simulink model "yolov9_orin_ipcam_detect"  (R2025b)
settings: Solver=FixedStepAuto  StartTime=0.0  StopTime=10.0  FixedStep=0.05  RelTol=1e-3
libraries: nvidiaAVlib x2
settings Model: Creator=The MathWorks, Inc.  LastModifiedBy=Dogukan.kilinc
settings SolverCC: AlgebraicLoopSolver=TrustRegion
settings OptimizationCC: EnhancedBackFolding=on  PassReuseOutputArgsAs=Individual arguments  ZeroExternalMemoryAtStartup=off  ZeroInternalMemoryAtStartup=off  InitFltsAndDblsToZero=on  MaxStackSize=64  OptimizeBlockOrder=speed  DifferentSizesBufferReuse=on  OptimizationCustomize=off  EfficientTunableParamExpr=on
settings DebuggingCC: ParameterTunabilityLossMsg=error  UnconnectedInputMsg=warning  UnconnectedOutputMsg=warning  UnconnectedLineMsg=warning
settings HardwareCC: ProdBitPerLong=64.0  ProdLargestAtomicInteger=LongLong  ProdLargestAtomicFloat=Double  ProdLongLongMode=on  ProdHWDeviceType=ARM Compatible->ARM 64-bit (LP64)  TargetLongLongMode=on
settings ModelReferenceCC: EnableRefExpFcnMdlSchedulingChecks=on
settings SFSimCC: UsePrecompiledLibraries=Avoid  SimTargetLang=C++  GPUAcceleration=on  SimGPUCompilerFlags=-DMW_TENSORRT_10  SimDLTargetLibrary=cudnn
settings RTWCC: SystemTargetFile=ert.tlc  HardwareBoard=NVIDIA Jetson  TLCOptions="-aInlineSetEventsForThisBaseRateFcn=TLC_FALSE -aSuppressMultiTaskScheduler=TLC_FALSE  -aRateBasedStepFcn=0"  TemplateMakefile=ert_default_tmf  Toolchain=NVCC for NVIDIA Embedded Processors  TargetLang=C++  GenerateGPUCode=CUDA  GPUComputeCapability=8.7  GPUCompilerFlags=-DMW_TENSORRT_10  DLTargetLibrary=tensorrt  DLDataType=fp16  DLArmComputeVersion=19.05  Description=Embedded Coder
settings CodeAppCC: ForceParamTrailComments=on  IgnoreCustomStorageClasses=off  OperatorAnnotations=on  SimulinkDataObjDesc=on  SFDataObjDesc=on  InsertBlockDesc=on
settings ERTTargetCC: InlinedParameterPlacement=Hierarchical  RootIOFormat=Structure reference  DynamicArrayContainerType=coder::array  CodeInterfacePackaging=C++ class  SubcomponentCodeInterfacePackaging=C++ class  SupportVariableSizeSignals=on

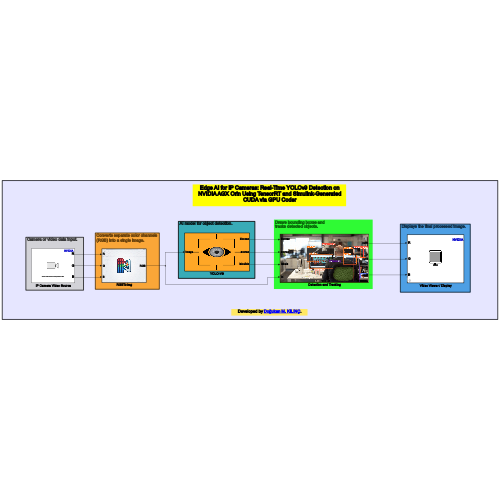
[thumbnail: Simulink's stored preview of the top-level diagram]
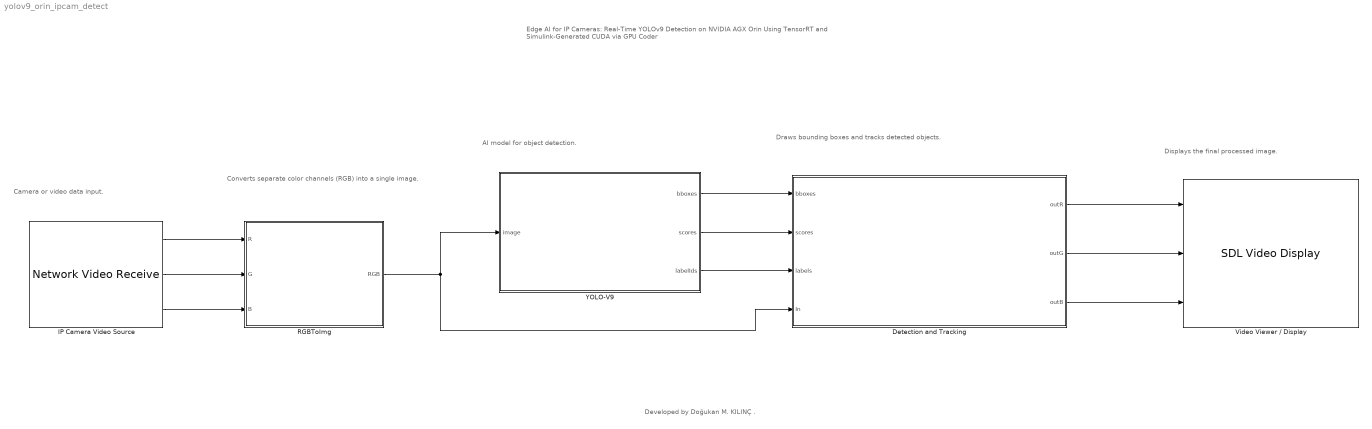
== system yolov9_orin_ipcam_detect ==
  note: Displays the final processed image.
  note: Camera or video data input.
  note: Converts separate color channels
      | (RGB) into a single image.
  note: Developed by Doğukan M. KILINÇ .
  note: Draws bounding boxes and
      | tracks detected objects.
  note: Edge AI for IP Cameras: Real-Time YOLOv9 Detection on NVIDIA AGX Orin Using TensorRT and Simulink-Generated CUDA via GPU Coder
  note: AI model for object detection.
  Detection and Tracking : MATLAB Function  TreatAsAtomicUnit=on
  IP Camera Video Source : nvidiaAVlib/Network Video Receive  VideoSource=Network device  ImageSize=[640, 480]  ImageFormat=Grayscale  DeviceImageFormat=RGB  DecoderFiltered=JPEG  ImageSignal=Separate color signal  Decoder=JPEG  VideoDeviceDecoder=H264  UseHWAcceleration=on  Port=3000  URI=rtsp://admin:admin1234@192.168.1.108:554/cam/realmonitor?channel=1&subtype=1  SampleTime=-1
  RGBToImg : MATLAB Function  TreatAsAtomicUnit=on
  Video Viewer / Display : nvidiaAVlib/SDL Video Display  pixelFormat=RGB  pixelOrder=Planar  rowMajor=on  blockPlatform=NVIDIA
  "YOLO-V9 " : MATLAB Function  TreatAsAtomicUnit=on
  wires:
  Detection and Tracking/1(outR) -> Video Viewer / Display/1
  IP Camera Video Source/2 -> RGBToImg/2(G)
  Detection and Tracking/3(outB) -> Video Viewer / Display/3
  "YOLO-V9 "/3(labelIds) -> Detection and Tracking/3(labels)
  "YOLO-V9 "/1(bboxes) -> Detection and Tracking/1(bboxes)
  IP Camera Video Source/3 -> RGBToImg/3(B)
  IP Camera Video Source/1 -> RGBToImg/1(R)
  "YOLO-V9 "/2(scores) -> Detection and Tracking/2(scores)
  RGBToImg/1(RGB) -> Detection and Tracking/4(In), "YOLO-V9 "/1(image)
  Detection and Tracking/2(outG) -> Video Viewer / Display/2

== matlab function yolov9_orin_ipcam_detect/YOLO-V9  ==
  data: image input size=[480, 640, 3]; bboxes output single size=[100, 4]; scores output single size=[100, 1]; labelIds output single size=[100, 1]
function [bboxes, scores, labelIds] = detectObjects(image)
    %#codegen
    
    % --- 1. INITIALIZATION AND MEMORY ALLOCATION ---
    % FIX 1: Assign default initial values to all output variables.
    % This prevents the Coder from throwing an "undefined" error.
    bboxes   = zeros(0, 4, 'single');
    scores   = zeros(0, 1, 'single');
    labelIds = zeros(0, 1, 'single');
    
    % --- 2. NETWORK LOADING (PERSISTENT MEMORY) ---
    persistent det
    if isempty(det)
        det = coder.loadDeepLearningNetwork('Yolov9t.mat');
    end
    
    % --- 3. INPUT DATA PREPROCESSING ---
    % Get the network input size.
    inputSize = [640 640 3];
    imgp = helper.preprocess(image, inputSize(1:2));
    
    % Convert the image to dlarray format.
    dlArray = dlarray(single(imgp), "SSCB");
    
    % --- 4. PREDICTION ---
    % Perform prediction using the loaded network (det) and dlArray.
    [out1, out2, out3] = predict(det, dlArray);
    
    % --- 5. OUTPUT RESTRUCTURING AND CONCATENATION ---
    % Perform post-processing on the network outputs.
    % Permute and reshape the outputs for further processing.
    out1p = permute(out1, [2 1 3 4]);
    out2p = permute(out2, [2 1 3 4]);
    out3p = permute(out3, [2 1 3 4]);
    
    out1r = reshape(out1p, [size(out1p, 1) * size(out1p, 2), size(out1p, 3)]);
    out2r = reshape(out2p, [size(out2p, 1) * size(out2p, 2), size(out2p, 3)]);
    out3r = reshape(out3p, [size(out3p, 1) * size(out3p, 2), size(out3p, 3)]);
    
    out = cat(1, out1r, out2r, out3r);
    
    % --- 6. MATLAB IMPLEMENTATION OF ONNX LAYERS ---
    % Post-processing implementation of ONNX layers in MATLAB.
    nclasses = 80;
    dim1 = size(out, 1);
    dim2 = size(out, 2);
    endIndex = dim2 - nclasses;
    
    splitout1 = out(:, 1:endIndex);
    splitout2 = out(:, endIndex+1:dim2);
    splitout2 = sigmoid(splitout2);
    
    splitout1 = reshape(splitout1, [dim1, 16, 4]);
    splitout1 = permute(splitout1, [1, 3, 2]);
    splitout1 = permute(splitout1, [2, 1, 3]);
    splitout1 = dlarray(single(splitout1), 'SSCB');
    
    softmaxout = softmax(splitout1);
    
    weights = dlarray(single(reshape(0:15, [1, 1, 16])));
    bias = dlarray(single(0));
    
    convout = dlconv(softmaxout, weights, bias);
    
    splitoutFc = extractdata(permute(convout, [2, 1, 3, 4]));
    splitoutFc = splitoutFc';
    
    split1 = single(splitoutFc(:, 1:2));
    split2 = single(splitoutFc(:, 3:4));
    
    % --- 7. ANCHOR AND STRIDE CALCULATIONS ---
    % Calculate constants for ONNX post-processing.
    sizes = [size(out1, 1) size(out1, 2); 
             size(out2, 1) size(out2, 2); 
             size(out3, 1) size(out3, 2)];
             
    % Constant 1
    ConstantArray1 = single(helper.generateCenters(sizes)); % Centers of the anchor boxes
    
    m1 = min((size(imgp, 1) / size(out1, 1)), (size(imgp, 2) / size(out1, 2)));
    m2 = min((size(imgp, 1) / size(out2, 1)), (size(imgp, 2) / size(out2, 2)));
    m3 = min((size(imgp, 1) / size(out3, 1)), (size(imgp, 2) / size(out3, 2)));
    
    Array2 = [repmat(m1, size(out1, 1)*size(out1, 2), 1); 
              repmat(m2, size(out2, 1)*size(out2, 2), 1); 
              repmat(m3, size(out3, 1)*size(out3, 2), 1)];
              
    % Constant 2
    ConstantArray2 = single(Array2); % Stride values
    
    % --- 8. BOUNDING BOX GENERATION ---
    % ONNX post-processing operations
    sub1 = ConstantArray1 - split1;
    add1 = ConstantArray1 + split2;
    add3 = sub1 + add1;
    div1 = add3 ./ 2;
    sub2 = add1 - sub1;
    
    concat1 = cat(2, div1, sub2);
    mul1 = concat1 .* ConstantArray2;
    mul1 = dlarray(mul1);
    
    combout = cat(2, mul1, splitout2);
    
    % Obtain the final feature map.
    featureMap = permute(combout, [2, 1]);
    
    % --- 9. FINAL PROCESSING AND OUTPUT LIMITATION ---
    % Apply post-processing on the extracted feature map.
    output = helper.postprocess(featureMap, imgp, image);
    
    % Step 1: STRICTLY prevent outputs from exceeding 100 detections
    numDetections = size(output, 1);
    n = min(numDetections, 100);
    
    if n > 0
        % Step 2: Extract data and STRICTLY cast to 'single' type.
        % (Prevents Simulink from losing types and mixing with uint8)
        bboxes   = single(output(1:n, 1:4));
        scores   = single(output(1:n, 5));
        labelIds = single(output(1:n, 6));
    else
        % If no detections, variables remain empty (initial allocations apply)
    end
end

== matlab function yolov9_orin_ipcam_detect/Detection and Tracking ==
  data: bboxes input; outR output; outG output; outB output; scores input single size=[100, 1]; labels input single size=[100, 1]; In input
function [outR, outG, outB] = detection_and_tracking(bboxes, scores, labels, In)
    %#codegen
    
    % --- 1. INITIALIZE OUTPUTS ---
    outR = zeros(640, 480, 'uint8');
    outG = zeros(640, 480, 'uint8');
    outB = zeros(640, 480, 'uint8');
    
    % --- 2. LOAD CLASS NAMES ---
    persistent classNames;
    if isempty(classNames)
        classNames = getCOCOClassNames();
    end
    
    % --- 3. START TIMER FOR FPS ---
    persistent averageFPS tStart;
    if isempty(averageFPS)
        averageFPS = single(0);
        tStart = tic; % Start the hardware timer
    end
    
    % --- 4. DRAW BOXES AND LABELS ---
    if ~isempty(bboxes)
        for i = 1:size(bboxes, 1)
            
            % Get the Class ID and Score
            classIndex = int32(labels(i));
            scoreVal   = int32(round(scores(i) * 100));
            
            % Guard check (Keep boundaries between 1-80)
            if classIndex < 1
                classIndex = int32(1);
            elseif classIndex > 80
                classIndex = int32(80);
            end
            
            % Extract the class name from the list
            cName = classNames{classIndex};
            
            % Create the text and overlay it on the image
            txt = sprintf('%s: %d%%', cName, scoreVal);
            In  = insertObjectAnnotation(In, 'rectangle', bboxes(i, :), txt);
        end
    end
    
    % --- 5. FPS CALCULATION AND DISPLAY ---
    % Measure elapsed time (in seconds)
    tElapsed = toc(tStart);
    
    % Safety check to prevent Divide by Zero errors
    if tElapsed > 0
        currentFPS = single(1.0 / tElapsed);
        
        % Moving Average (Smoothed FPS calculation: 90% old average, 10% new instantaneous value)
        if averageFPS == 0
            averageFPS = currentFPS; % Assign directly on the first frame
        else
            averageFPS = (0.9 * averageFPS) + (0.1 * currentFPS);
        end
    end
    
    % Reset the timer for the next frame
    tStart = tic;
    
    % Create the FPS text and display it at the top-left corner (e.g., at coordinates [10, 20])
    fpsTxt = sprintf('FPS: %0.1f', averageFPS);
    In = insertText(In, [10, 20], fpsTxt, 'FontSize', 22, 'BoxColor', 'black', 'TextColor', 'white');
    
    % --- 6. CHANNEL SEPARATION AND TRANSPOSE (OUTPUT FORMATTING) ---
    outR(:, :) = In(:, :, 1)';
    outG(:, :) = In(:, :, 2)';
    outB(:, :) = In(:, :, 3)';
end

== matlab function yolov9_orin_ipcam_detect/RGBToImg ==
  data: R input; RGB output; G input; B input
function RGB = rgb2Img(R,G,B)
    % Swapping dimensions from the NVIDIA camera for MATLAB (Width <-> Height)
    RGB = coder.nullcopy(uint8(zeros(size(R,2), size(R,1), 3)));
    
    % Transposing (') the R, G, and B channels to correct the image
    RGB(:,:,1) = R';
    RGB(:,:,2) = G';
    RGB(:,:,3) = B';
end
